simulink model "PrkgClimaPreProcMgr"  (R2022b) (continued)
== system PrkgClimaPreProcMgr == (continued)
  ClngRqrdFromTelmConnGen2_ClngRqrdFromTelmConnGen2/1 -> PrkgClimaPreProcMgr_Runnable_sys/17(ClngRqrdFromTelmConnGen2_ClngRqrdFromTelmConnGen2_read)
  CmptmtObsvrSts_CmptmtObsvrSts/1 -> PrkgClimaPreProcMgr_Runnable_sys/18(CmptmtObsvrSts_CmptmtObsvrSts_read)
  CmptmtSunMtrlTEstimd_CmptmtSunMtrlTEstimd/1 -> PrkgClimaPreProcMgr_Runnable_sys/19(CmptmtSunMtrlTEstimd_CmptmtSunMtrlTEstimd_read)
  DCChrgnHndlSts_DCChrgnHndlSts/1 -> PrkgClimaPreProcMgr_Runnable_sys/20(DCChrgnHndlSts_DCChrgnHndlSts_read)
  DshbTForClima_DshbTForClima/1 -> PrkgClimaPreProcMgr_Runnable_sys/21(DshbTForClima_DshbTForClima_read)
  DshbTForClimaQf_DshbTForClimaQf/1 -> PrkgClimaPreProcMgr_Runnable_sys/22(DshbTForClimaQf_DshbTForClimaQf_read)
  HvacAirTForRowFirstAtVentLeRaw_HvacAirTForRowFirstAtVentLeRaw/1 -> PrkgClimaPreProcMgr_Runnable_sys/23(HvacAirTForRowFirstAtVentLeRaw_HvacAirTForRowFirstAtVentLeRaw_read)
  HvacAirTForRowFirstAtVentRiRaw_HvacAirTForRowFirstAtVentRiRaw/1 -> PrkgClimaPreProcMgr_Runnable_sys/24(HvacAirTForRowFirstAtVentRiRaw_HvacAirTForRowFirstAtVentRiRaw_read)
  MemReadForPrkgClimaPreProcMgr_MemReadForPrkgClimaPreProcMgr/1 -> PrkgClimaPreProcMgr_Runnable_sys/25(MemReadForPrkgClimaPreProcMgr_MemReadForPrkgClimaPreProcMgr_read)
  MemReqStsForPrkgClima_MemReqStsForPrkgClima/1 -> PrkgClimaPreProcMgr_Runnable_sys/26(MemReqStsForPrkgClima_MemReqStsForPrkgClima_read)
  NetReqFromPrkgClimaEveMgr_NetReqFromPrkgClimaEveMgr/1 -> PrkgClimaPreProcMgr_Runnable_sys/27(NetReqFromPrkgClimaEveMgr_NetReqFromPrkgClimaEveMgr_read)
  OnBdChrgrHndlSts_OnBdChrgrHndlSts/1 -> PrkgClimaPreProcMgr_Runnable_sys/28(OnBdChrgrHndlSts_OnBdChrgrHndlSts_read)
  ReqForPrkgClima_ReqForPrkgClima/1 -> PrkgClimaPreProcMgr_Runnable_sys/29(ReqForPrkgClima_ReqForPrkgClima_read)
  ReqForSwtHeatrTypFromActvMgr_ReqForSwtHeatrTypFromActvMgr/1 -> PrkgClimaPreProcMgr_Runnable_sys/30(ReqForSwtHeatrTypFromActvMgr_ReqForSwtHeatrTypFromActvMgr_read)
  ReqForSwtHeatrTypFromEveMgr_ReqForSwtHeatrTypFromEveMgr/1 -> PrkgClimaPreProcMgr_Runnable_sys/31(ReqForSwtHeatrTypFromEveMgr_ReqForSwtHeatrTypFromEveMgr_read)
  RtMaxForPrePrkgClima_RtMaxForPrePrkgClima/1 -> PrkgClimaPreProcMgr_Runnable_sys/32(RtMaxForPrePrkgClima_RtMaxForPrePrkgClima_read)
  SeatHeatDurgClimaEnadConnGen2_SeatHeatDurgClimaEnadConnGen2/1 -> PrkgClimaPreProcMgr_Runnable_sys/33(SeatHeatDurgClimaEnadConnGen2_SeatHeatDurgClimaEnadConnGen2_read)
  SteerWhlHeatgDurgClimEandFromTelmConnGen2_SteerWhlHeatgDurgClimEandFromTelmConnGen2/1 -> PrkgClimaPreProcMgr_Runnable_sys/34(SteerWhlHeatgDurgClimEandFromTelmConnGen2_SteerWhlHeatgDurgClimEandFromTelmConnGen2_read)
  VehModMngtGlbSafe1_Ngcc_VehModMngtGlbSafe1/1 -> PrkgClimaPreProcMgr_Runnable_sys/35(VehModMngtGlbSafe1_Ngcc_VehModMngtGlbSafe1_read)
  WarnForEgyAllwdLimd_WarnForEgyAllwdLimd/1 -> PrkgClimaPreProcMgr_Runnable_sys/36(WarnForEgyAllwdLimd_WarnForEgyAllwdLimd_read)
  WarnForHybWoUForTmr_WarnForHybWoUForTmr/1 -> PrkgClimaPreProcMgr_Runnable_sys/37(WarnForHybWoUForTmr_WarnForHybWoUForTmr_read)
  WarnForHybWoUFromActvMgr_WarnForHybWoUFromActvMgr/1 -> PrkgClimaPreProcMgr_Runnable_sys/38(WarnForHybWoUFromActvMgr_WarnForHybWoUFromActvMgr_read)
  WarnForTmrPreCdngFctNotAvl_WarnForTmrPreCdngFctNotAvl/1 -> PrkgClimaPreProcMgr_Runnable_sys/39(WarnForTmrPreCdngFctNotAvl_WarnForTmrPreCdngFctNotAvl_read)
  BookTrvlClimaActvReq_Ngcc_BookTrvlClimaActvReq/1 -> PrkgClimaPreProcMgr_Runnable_sys/40(BookTrvlClimaActvReq_Ngcc_BookTrvlClimaActvReq_read)
  BookTrvlClimaActvReq_Ngcc_BookTrvlClimaActvReq_IsUpdated/1 -> PrkgClimaPreProcMgr_Runnable_sys/41(BookTrvlClimaActvReq_Ngcc_BookTrvlClimaActvReq_IsUpdated_read)
  CbnOverHeatProtnEna_CbnOverHeatProtnEna/1 -> PrkgClimaPreProcMgr_Runnable_sys/42(CbnOverHeatProtnEna_CbnOverHeatProtnEna_read)
  CbnOverHeatProtnEna_CbnOverHeatProtnEna_IsUpdated/1 -> PrkgClimaPreProcMgr_Runnable_sys/43(CbnOverHeatProtnEna_CbnOverHeatProtnEna_IsUpdated_read)
  CbnOverHeatProtnEnaFromTelm_CbnOverHeatProtnEnaFromTelm/1 -> PrkgClimaPreProcMgr_Runnable_sys/44(CbnOverHeatProtnEnaFromTelm_CbnOverHeatProtnEnaFromTelm_read)
  CbnOverHeatProtnEnaFromTelm_CbnOverHeatProtnEnaFromTelm_IsUpdated/1 -> PrkgClimaPreProcMgr_Runnable_sys/45(CbnOverHeatProtnEnaFromTelm_CbnOverHeatProtnEnaFromTelm_IsUpdated_read)
  CdsClimaOnReq_CdsClimaOnReq/1 -> PrkgClimaPreProcMgr_Runnable_sys/46(CdsClimaOnReq_CdsClimaOnReq_read)
  ClimaRqrdFromHmi_ClimaRqrdFromHmi/1 -> PrkgClimaPreProcMgr_Runnable_sys/47(ClimaRqrdFromHmi_ClimaRqrdFromHmi_read)
  ClimaRqrdFromHmi_ClimaRqrdFromHmi_IsUpdated/1 -> PrkgClimaPreProcMgr_Runnable_sys/48(ClimaRqrdFromHmi_ClimaRqrdFromHmi_IsUpdated_read)
  ClimaTmr_ClimaTmr/1 -> PrkgClimaPreProcMgr_Runnable_sys/49(ClimaTmr_ClimaTmr_read)
  ClimaTmr_ClimaTmr_IsUpdated/1 -> PrkgClimaPreProcMgr_Runnable_sys/50(ClimaTmr_ClimaTmr_IsUpdated_read)
  ClimaTmrStsRqrd_ClimaTmrStsRqrd/1 -> PrkgClimaPreProcMgr_Runnable_sys/51(ClimaTmrStsRqrd_ClimaTmrStsRqrd_read)
  ClimaTmrStsRqrd_ClimaTmrStsRqrd_IsUpdated/1 -> PrkgClimaPreProcMgr_Runnable_sys/52(ClimaTmrStsRqrd_ClimaTmrStsRqrd_IsUpdated_read)
  ClngRqrdFromHmi_ClngRqrdFromHmi/1 -> PrkgClimaPreProcMgr_Runnable_sys/53(ClngRqrdFromHmi_ClngRqrdFromHmi_read)
  ClngRqrdFromHmi_ClngRqrdFromHmi_IsUpdated/1 -> PrkgClimaPreProcMgr_Runnable_sys/54(ClngRqrdFromHmi_ClngRqrdFromHmi_IsUpdated_read)
  ClngRqrdFromTelm_ClngRqrdFromTelm/1 -> PrkgClimaPreProcMgr_Runnable_sys/55(ClngRqrdFromTelm_ClngRqrdFromTelm_read)
  ClngRqrdFromTelm_ClngRqrdFromTelm_IsUpdated/1 -> PrkgClimaPreProcMgr_Runnable_sys/56(ClngRqrdFromTelm_ClngRqrdFromTelm_IsUpdated_read)
  ErsCmdByKey_ErsCmdByKey/1 -> PrkgClimaPreProcMgr_Runnable_sys/57(ErsCmdByKey_ErsCmdByKey_read)
  ErsCmdByKey_ErsCmdByKey_IsUpdated/1 -> PrkgClimaPreProcMgr_Runnable_sys/58(ErsCmdByKey_ErsCmdByKey_IsUpdated_read)
  HeatrPreCdngTyp_HeatrPreCdngTyp/1 -> PrkgClimaPreProcMgr_Runnable_sys/59(HeatrPreCdngTyp_HeatrPreCdngTyp_read)
  HeatrPreCdngTyp_HeatrPreCdngTyp_IsUpdated/1 -> PrkgClimaPreProcMgr_Runnable_sys/60(HeatrPreCdngTyp_HeatrPreCdngTyp_IsUpdated_read)
  IncomingCO2CtrlFromHmi_IncomingCO2CtrlFromHmi/1 -> PrkgClimaPreProcMgr_Runnable_sys/61(IncomingCO2CtrlFromHmi_IncomingCO2CtrlFromHmi_read)
  IncomingCO2CtrlFromHmi_IncomingCO2CtrlFromHmi_IsUpdated/1 -> PrkgClimaPreProcMgr_Runnable_sys/62(IncomingCO2CtrlFromHmi_IncomingCO2CtrlFromHmi_IsUpdated_read)
  RemStrtHvCtrlReq_Ngcc_RemStrtHvCtrlReq/1 -> PrkgClimaPreProcMgr_Runnable_sys/63(RemStrtHvCtrlReq_Ngcc_RemStrtHvCtrlReq_read)
  RemStrtHvCtrlReq_Ngcc_RemStrtHvCtrlReq_IsUpdated/1 -> PrkgClimaPreProcMgr_Runnable_sys/64(RemStrtHvCtrlReq_Ngcc_RemStrtHvCtrlReq_IsUpdated_read)
  RemVentReq_RemVentReq/1 -> PrkgClimaPreProcMgr_Runnable_sys/65(RemVentReq_RemVentReq_read)
  RemVentReq_RemVentReq_IsUpdated/1 -> PrkgClimaPreProcMgr_Runnable_sys/66(RemVentReq_RemVentReq_IsUpdated_read)
  SeatHeatDurgClimaEnad_SeatHeatDurgClimaEnad/1 -> PrkgClimaPreProcMgr_Runnable_sys/67(SeatHeatDurgClimaEnad_SeatHeatDurgClimaEnad_read)
  SeatHeatDurgClimaEnad_SeatHeatDurgClimaEnad_IsUpdated/1 -> PrkgClimaPreProcMgr_Runnable_sys/68(SeatHeatDurgClimaEnad_SeatHeatDurgClimaEnad_IsUpdated_read)
  SeatHeatDurgClimaEnadFromTelm_Ngcc_SeatHeatDurgClimaEnadFromTelm/1 -> PrkgClimaPreProcMgr_Runnable_sys/69(SeatHeatDurgClimaEnadFromTelm_Ngcc_SeatHeatDurgClimaEnadFromTelm_read)
  SeatHeatDurgClimaEnadFromTelm_Ngcc_SeatHeatDurgClimaEnadFromTelm_IsUpdated/1 -> PrkgClimaPreProcMgr_Runnable_sys/70(SeatHeatDurgClimaEnadFromTelm_Ngcc_SeatHeatDurgClimaEnadFromTelm_IsUpdated_read)
  SteerWhlHeatgDurgClimaEnad_SteerWhlHeatgDurgClimaEnad/1 -> PrkgClimaPreProcMgr_Runnable_sys/71(SteerWhlHeatgDurgClimaEnad_SteerWhlHeatgDurgClimaEnad_read)
  SteerWhlHeatgDurgClimaEnad_SteerWhlHeatgDurgClimaEnad_IsUpdated/1 -> PrkgClimaPreProcMgr_Runnable_sys/72(SteerWhlHeatgDurgClimaEnad_SteerWhlHeatgDurgClimaEnad_IsUpdated_read)
  SteerWhlHeatgDurgClimaEnadFromTelm_SteerWhlHeatgDurgClimaEnadFromTelm/1 -> PrkgClimaPreProcMgr_Runnable_sys/73(SteerWhlHeatgDurgClimaEnadFromTelm_SteerWhlHeatgDurgClimaEnadFromTelm_read)
  SteerWhlHeatgDurgClimaEnadFromTelm_SteerWhlHeatgDurgClimaEnadFromTelm_IsUpdated/1 -> PrkgClimaPreProcMgr_Runnable_sys/74(SteerWhlHeatgDurgClimaEnadFromTelm_SteerWhlHeatgDurgClimaEnadFromTelm_IsUpdated_read)
  StrtRemSts1WdStrtRemSts_Ngcc_StrtRemSts1WdStrtRemSts/1 -> PrkgClimaPreProcMgr_Runnable_sys/75(StrtRemSts1WdStrtRemSts_Ngcc_StrtRemSts1WdStrtRemSts_read)
  StrtRemSts1WdStrtRemSts_Ngcc_StrtRemSts1WdStrtRemSts_IsUpdated/1 -> PrkgClimaPreProcMgr_Runnable_sys/76(StrtRemSts1WdStrtRemSts_Ngcc_StrtRemSts1WdStrtRemSts_IsUpdated_read)
  TelmClimaReq_TelmClimaReq/1 -> PrkgClimaPreProcMgr_Runnable_sys/77(TelmClimaReq_TelmClimaReq_read)
  TelmClimaReq_TelmClimaReq_IsUpdated/1 -> PrkgClimaPreProcMgr_Runnable_sys/78(TelmClimaReq_TelmClimaReq_IsUpdated_read)
  TelmClimaTmr_TelmClimaTmr/1 -> PrkgClimaPreProcMgr_Runnable_sys/79(TelmClimaTmr_TelmClimaTmr_read)
  TelmClimaTmr_TelmClimaTmr_IsUpdated/1 -> PrkgClimaPreProcMgr_Runnable_sys/80(TelmClimaTmr_TelmClimaTmr_IsUpdated_read)
  TelmClimaTSet_TelmClimaTSet/1 -> PrkgClimaPreProcMgr_Runnable_sys/81(TelmClimaTSet_TelmClimaTSet_read)
  TelmClimaTSet_TelmClimaTSet_IsUpdated/1 -> PrkgClimaPreProcMgr_Runnable_sys/82(TelmClimaTSet_TelmClimaTSet_IsUpdated_read)
  TelmSeatDrvHeatClimaLvl_TelmSeatDrvHeatClimaLvl/1 -> PrkgClimaPreProcMgr_Runnable_sys/83(TelmSeatDrvHeatClimaLvl_TelmSeatDrvHeatClimaLvl_read)
  TelmSeatDrvHeatClimaLvl_TelmSeatDrvHeatClimaLvl_IsUpdated/1 -> PrkgClimaPreProcMgr_Runnable_sys/84(TelmSeatDrvHeatClimaLvl_TelmSeatDrvHeatClimaLvl_IsUpdated_read)
  TelmSeatDrvVentnClimaLvl_TelmSeatDrvVentnClimaLvl/1 -> PrkgClimaPreProcMgr_Runnable_sys/85(TelmSeatDrvVentnClimaLvl_TelmSeatDrvVentnClimaLvl_read)
  TelmSeatDrvVentnClimaLvl_TelmSeatDrvVentnClimaLvl_IsUpdated/1 -> PrkgClimaPreProcMgr_Runnable_sys/86(TelmSeatDrvVentnClimaLvl_TelmSeatDrvVentnClimaLvl_IsUpdated_read)
  TelmSeatPassHeatClimaLvl_TelmSeatPassHeatClimaLvl/1 -> PrkgClimaPreProcMgr_Runnable_sys/87(TelmSeatPassHeatClimaLvl_TelmSeatPassHeatClimaLvl_read)
  TelmSeatPassHeatClimaLvl_TelmSeatPassHeatClimaLvl_IsUpdated/1 -> PrkgClimaPreProcMgr_Runnable_sys/88(TelmSeatPassHeatClimaLvl_TelmSeatPassHeatClimaLvl_IsUpdated_read)
  TelmSeatPassVentnClimaLvl_TelmSeatPassVentnClimaLvl/1 -> PrkgClimaPreProcMgr_Runnable_sys/89(TelmSeatPassVentnClimaLvl_TelmSeatPassVentnClimaLvl_read)
  TelmSeatPassVentnClimaLvl_TelmSeatPassVentnClimaLvl_IsUpdated/1 -> PrkgClimaPreProcMgr_Runnable_sys/90(TelmSeatPassVentnClimaLvl_TelmSeatPassVentnClimaLvl_IsUpdated_read)
  TelmSeatSecLeHeatClimaLvl_TelmSeatSecLeHeatClimaLvl/1 -> PrkgClimaPreProcMgr_Runnable_sys/91(TelmSeatSecLeHeatClimaLvl_TelmSeatSecLeHeatClimaLvl_read)
  TelmSeatSecLeHeatClimaLvl_TelmSeatSecLeHeatClimaLvl_IsUpdated/1 -> PrkgClimaPreProcMgr_Runnable_sys/92(TelmSeatSecLeHeatClimaLvl_TelmSeatSecLeHeatClimaLvl_IsUpdated_read)
  TelmSeatSecLeVentnClimaLvl_TelmSeatSecLeVentnClimaLvl/1 -> PrkgClimaPreProcMgr_Runnable_sys/93(TelmSeatSecLeVentnClimaLvl_TelmSeatSecLeVentnClimaLvl_read)
  TelmSeatSecLeVentnClimaLvl_TelmSeatSecLeVentnClimaLvl_IsUpdated/1 -> PrkgClimaPreProcMgr_Runnable_sys/94(TelmSeatSecLeVentnClimaLvl_TelmSeatSecLeVentnClimaLvl_IsUpdated_read)
  TelmSeatSecRiHeatClimaLvl_TelmSeatSecRiHeatClimaLvl/1 -> PrkgClimaPreProcMgr_Runnable_sys/95(TelmSeatSecRiHeatClimaLvl_TelmSeatSecRiHeatClimaLvl_read)
  TelmSeatSecRiHeatClimaLvl_TelmSeatSecRiHeatClimaLvl_IsUpdated/1 -> PrkgClimaPreProcMgr_Runnable_sys/96(TelmSeatSecRiHeatClimaLvl_TelmSeatSecRiHeatClimaLvl_IsUpdated_read)
  TelmSeatSecRiVentnClimaLvl_TelmSeatSecRiVentnClimaLvl/1 -> PrkgClimaPreProcMgr_Runnable_sys/97(TelmSeatSecRiVentnClimaLvl_TelmSeatSecRiVentnClimaLvl_read)
  TelmSeatSecRiVentnClimaLvl_TelmSeatSecRiVentnClimaLvl_IsUpdated/1 -> PrkgClimaPreProcMgr_Runnable_sys/98(TelmSeatSecRiVentnClimaLvl_TelmSeatSecRiVentnClimaLvl_IsUpdated_read)
  HMIExtVent_HMIExtVent/1 -> PrkgClimaPreProcMgr_Runnable_sys/99(HMIExtVent_HMIExtVent_read)
  HMIExtVent_HMIExtVent_IsUpdated/1 -> PrkgClimaPreProcMgr_Runnable_sys/100(HMIExtVent_HMIExtVent_IsUpdated_read)
  BookTrvlClimaActvReq2_BookTrvlClimaActvReq2/1 -> PrkgClimaPreProcMgr_Runnable_sys/101(BookTrvlClimaActvReq2_BookTrvlClimaActvReq2_read)
  BookTrvlClimaActvReq2_BookTrvlClimaActvReq2_IsUpdated/1 -> PrkgClimaPreProcMgr_Runnable_sys/102(BookTrvlClimaActvReq2_BookTrvlClimaActvReq2_IsUpdated_read)
  BookTrvlClimaActvReq3_BookTrvlClimaActvReq3/1 -> PrkgClimaPreProcMgr_Runnable_sys/103(BookTrvlClimaActvReq3_BookTrvlClimaActvReq3_read)
  BookTrvlClimaActvReq3_BookTrvlClimaActvReq3_IsUpdated/1 -> PrkgClimaPreProcMgr_Runnable_sys/104(BookTrvlClimaActvReq3_BookTrvlClimaActvReq3_IsUpdated_read)
  BookTrvlClimaActvReq4_BookTrvlClimaActvReq4/1 -> PrkgClimaPreProcMgr_Runnable_sys/105(BookTrvlClimaActvReq4_BookTrvlClimaActvReq4_read)
  BookTrvlClimaActvReq4_BookTrvlClimaActvReq4_IsUpdated/1 -> PrkgClimaPreProcMgr_Runnable_sys/106(BookTrvlClimaActvReq4_BookTrvlClimaActvReq4_IsUpdated_read)
  BookTrvlClimaActvReq5_BookTrvlClimaActvReq5/1 -> PrkgClimaPreProcMgr_Runnable_sys/107(BookTrvlClimaActvReq5_BookTrvlClimaActvReq5_read)
  BookTrvlClimaActvReq5_BookTrvlClimaActvReq5_IsUpdated/1 -> PrkgClimaPreProcMgr_Runnable_sys/108(BookTrvlClimaActvReq5_BookTrvlClimaActvReq5_IsUpdated_read)
  BookTrvlClimaActvReq6_BookTrvlClimaActvReq6/1 -> PrkgClimaPreProcMgr_Runnable_sys/109(BookTrvlClimaActvReq6_BookTrvlClimaActvReq6_read)
  BookTrvlClimaActvReq6_BookTrvlClimaActvReq6_IsUpdated/1 -> PrkgClimaPreProcMgr_Runnable_sys/110(BookTrvlClimaActvReq6_BookTrvlClimaActvReq6_IsUpdated_read)
  BookTrvlClimaActvReq7_BookTrvlClimaActvReq7/1 -> PrkgClimaPreProcMgr_Runnable_sys/111(BookTrvlClimaActvReq7_BookTrvlClimaActvReq7_read)
  BookTrvlClimaActvReq7_BookTrvlClimaActvReq7_IsUpdated/1 -> PrkgClimaPreProcMgr_Runnable_sys/112(BookTrvlClimaActvReq7_BookTrvlClimaActvReq7_IsUpdated_read)
  ClimaWakUpTiDif1_ClimaWakUpTiDif1/1 -> PrkgClimaPreProcMgr_Runnable_sys/113(ClimaWakUpTiDif1_ClimaWakUpTiDif1_read)
  ClimaWakUpTiDif1_ClimaWakUpTiDif1_IsUpdated/1 -> PrkgClimaPreProcMgr_Runnable_sys/114(ClimaWakUpTiDif1_ClimaWakUpTiDif1_IsUpdated_read)
  ClimaWakUpTiDif2_ClimaWakUpTiDif2/1 -> PrkgClimaPreProcMgr_Runnable_sys/115(ClimaWakUpTiDif2_ClimaWakUpTiDif2_read)
  ClimaWakUpTiDif2_ClimaWakUpTiDif2_IsUpdated/1 -> PrkgClimaPreProcMgr_Runnable_sys/116(ClimaWakUpTiDif2_ClimaWakUpTiDif2_IsUpdated_read)
  ClimaWakUpTiDif3_ClimaWakUpTiDif3/1 -> PrkgClimaPreProcMgr_Runnable_sys/117(ClimaWakUpTiDif3_ClimaWakUpTiDif3_read)
  ClimaWakUpTiDif3_ClimaWakUpTiDif3_IsUpdated/1 -> PrkgClimaPreProcMgr_Runnable_sys/118(ClimaWakUpTiDif3_ClimaWakUpTiDif3_IsUpdated_read)
  ClimaWakUpTiDif4_ClimaWakUpTiDif4/1 -> PrkgClimaPreProcMgr_Runnable_sys/119(ClimaWakUpTiDif4_ClimaWakUpTiDif4_read)
  ClimaWakUpTiDif4_ClimaWakUpTiDif4_IsUpdated/1 -> PrkgClimaPreProcMgr_Runnable_sys/120(ClimaWakUpTiDif4_ClimaWakUpTiDif4_IsUpdated_read)
  ClimaWakUpTiDif5_ClimaWakUpTiDif5/1 -> PrkgClimaPreProcMgr_Runnable_sys/121(ClimaWakUpTiDif5_ClimaWakUpTiDif5_read)
  ClimaWakUpTiDif5_ClimaWakUpTiDif5_IsUpdated/1 -> PrkgClimaPreProcMgr_Runnable_sys/122(ClimaWakUpTiDif5_ClimaWakUpTiDif5_IsUpdated_read)
  ClimaWakUpTiDif6_ClimaWakUpTiDif6/1 -> PrkgClimaPreProcMgr_Runnable_sys/123(ClimaWakUpTiDif6_ClimaWakUpTiDif6_read)
  ClimaWakUpTiDif6_ClimaWakUpTiDif6_IsUpdated/1 -> PrkgClimaPreProcMgr_Runnable_sys/124(ClimaWakUpTiDif6_ClimaWakUpTiDif6_IsUpdated_read)
  ClimaWakUpTiDif7_ClimaWakUpTiDif7/1 -> PrkgClimaPreProcMgr_Runnable_sys/125(ClimaWakUpTiDif7_ClimaWakUpTiDif7_read)
  ClimaWakUpTiDif7_ClimaWakUpTiDif7_IsUpdated/1 -> PrkgClimaPreProcMgr_Runnable_sys/126(ClimaWakUpTiDif7_ClimaWakUpTiDif7_IsUpdated_read)
  EngSt1WdSts_Ngcc_EngSt1WdSts/1 -> PrkgClimaPreProcMgr_Runnable_sys/127(EngSt1WdSts_Ngcc_EngSt1WdSts_read)
  HMIExtVentonoffReq_HMIExtVentonoffReq/1 -> PrkgClimaPreProcMgr_Runnable_sys/128(HMIExtVentonoffReq_HMIExtVentonoffReq_read)
  HMIExtVentonoffReq_HMIExtVentonoffReq_IsUpdated/1 -> PrkgClimaPreProcMgr_Runnable_sys/129(HMIExtVentonoffReq_HMIExtVentonoffReq_IsUpdated_read)
  RemStrtExtnTiReq_RemStrtExtnTiReq/1 -> PrkgClimaPreProcMgr_Runnable_sys/130(RemStrtExtnTiReq_RemStrtExtnTiReq_read)
  RemStrtExtnTiReq_RemStrtExtnTiReq_IsUpdated/1 -> PrkgClimaPreProcMgr_Runnable_sys/131(RemStrtExtnTiReq_RemStrtExtnTiReq_IsUpdated_read)
  TelmBookClimaReq_TelmBookClimaReq/1 -> PrkgClimaPreProcMgr_Runnable_sys/132(TelmBookClimaReq_TelmBookClimaReq_read)
  TelmBookClimaReq_TelmBookClimaReq_IsUpdated/1 -> PrkgClimaPreProcMgr_Runnable_sys/133(TelmBookClimaReq_TelmBookClimaReq_IsUpdated_read)
  TelmBookClimaTmr_TelmBookClimaTmr/1 -> PrkgClimaPreProcMgr_Runnable_sys/134(TelmBookClimaTmr_TelmBookClimaTmr_read)
  TelmBookClimaTmr_TelmBookClimaTmr_IsUpdated/1 -> PrkgClimaPreProcMgr_Runnable_sys/135(TelmBookClimaTmr_TelmBookClimaTmr_IsUpdated_read)
  TelmBookSeatHeatClimalReq_TelmBookSeatHeatClimalReq/1 -> PrkgClimaPreProcMgr_Runnable_sys/136(TelmBookSeatHeatClimalReq_TelmBookSeatHeatClimalReq_read)
  TelmBookSeatHeatClimalReq_TelmBookSeatHeatClimalReq_IsUpdated/1 -> PrkgClimaPreProcMgr_Runnable_sys/137(TelmBookSeatHeatClimalReq_TelmBookSeatHeatClimalReq_IsUpdated_read)
  TelmBookSeatVentnClimalReq_TelmBookSeatVentnClimalReq/1 -> PrkgClimaPreProcMgr_Runnable_sys/138(TelmBookSeatVentnClimalReq_TelmBookSeatVentnClimalReq_read)
  TelmBookSeatVentnClimalReq_TelmBookSeatVentnClimalReq_IsUpdated/1 -> PrkgClimaPreProcMgr_Runnable_sys/139(TelmBookSeatVentnClimalReq_TelmBookSeatVentnClimalReq_IsUpdated_read)
  TelmBookSteerWhlHeatClimalReq_TelmBookSteerWhlHeatClimalReq/1 -> PrkgClimaPreProcMgr_Runnable_sys/140(TelmBookSteerWhlHeatClimalReq_TelmBookSteerWhlHeatClimalReq_read)
  TelmBookSteerWhlHeatClimalReq_TelmBookSteerWhlHeatClimalReq_IsUpdated/1 -> PrkgClimaPreProcMgr_Runnable_sys/141(TelmBookSteerWhlHeatClimalReq_TelmBookSteerWhlHeatClimalReq_IsUpdated_read)
  TelmClimaExtVentnLvl_TelmClimaExtVentnLvl/1 -> PrkgClimaPreProcMgr_Runnable_sys/142(TelmClimaExtVentnLvl_TelmClimaExtVentnLvl_read)
  TelmClimaExtVentnLvl_TelmClimaExtVentnLvl_IsUpdated/1 -> PrkgClimaPreProcMgr_Runnable_sys/143(TelmClimaExtVentnLvl_TelmClimaExtVentnLvl_IsUpdated_read)
  TelmClimaExtVentnReq_TelmClimaExtVentnReq/1 -> PrkgClimaPreProcMgr_Runnable_sys/144(TelmClimaExtVentnReq_TelmClimaExtVentnReq_read)
  TelmClimaExtVentnReq_TelmClimaExtVentnReq_IsUpdated/1 -> PrkgClimaPreProcMgr_Runnable_sys/145(TelmClimaExtVentnReq_TelmClimaExtVentnReq_IsUpdated_read)
  TelmClimaExtVentnTempSet_TelmClimaExtVentnTempSet/1 -> PrkgClimaPreProcMgr_Runnable_sys/146(TelmClimaExtVentnTempSet_TelmClimaExtVentnTempSet_read)
  TelmClimaExtVentnTempSet_TelmClimaExtVentnTempSet_IsUpdated/1 -> PrkgClimaPreProcMgr_Runnable_sys/147(TelmClimaExtVentnTempSet_TelmClimaExtVentnTempSet_IsUpdated_read)
  TelmDefrostReq_TelmDefrostReq/1 -> PrkgClimaPreProcMgr_Runnable_sys/148(TelmDefrostReq_TelmDefrostReq_read)
  TelmDefrostReq_TelmDefrostReq_IsUpdated/1 -> PrkgClimaPreProcMgr_Runnable_sys/149(TelmDefrostReq_TelmDefrostReq_IsUpdated_read)
  TelmOxygenReq_TelmOxygenReq/1 -> PrkgClimaPreProcMgr_Runnable_sys/150(TelmOxygenReq_TelmOxygenReq_read)
  TelmOxygenReq_TelmOxygenReq_IsUpdated/1 -> PrkgClimaPreProcMgr_Runnable_sys/151(TelmOxygenReq_TelmOxygenReq_IsUpdated_read)
  TelmSeatThrdLeHeatClimaLvl_TelmSeatThrdLeHeatClimaLvl/1 -> PrkgClimaPreProcMgr_Runnable_sys/152(TelmSeatThrdLeHeatClimaLvl_TelmSeatThrdLeHeatClimaLvl_read)
  TelmSeatThrdLeHeatClimaLvl_TelmSeatThrdLeHeatClimaLvl_IsUpdated/1 -> PrkgClimaPreProcMgr_Runnable_sys/153(TelmSeatThrdLeHeatClimaLvl_TelmSeatThrdLeHeatClimaLvl_IsUpdated_read)
  TelmSeatThrdLeVentnClimaLvl_TelmSeatThrdLeVentnClimaLvl/1 -> PrkgClimaPreProcMgr_Runnable_sys/154(TelmSeatThrdLeVentnClimaLvl_TelmSeatThrdLeVentnClimaLvl_read)
  TelmSeatThrdLeVentnClimaLvl_TelmSeatThrdLeVentnClimaLvl_IsUpdated/1 -> PrkgClimaPreProcMgr_Runnable_sys/155(TelmSeatThrdLeVentnClimaLvl_TelmSeatThrdLeVentnClimaLvl_IsUpdated_read)
  TelmSeatThrdRiHeatClimaLvl_TelmSeatThrdRiHeatClimaLvl/1 -> PrkgClimaPreProcMgr_Runnable_sys/156(TelmSeatThrdRiHeatClimaLvl_TelmSeatThrdRiHeatClimaLvl_read)
  TelmSeatThrdRiHeatClimaLvl_TelmSeatThrdRiHeatClimaLvl_IsUpdated/1 -> PrkgClimaPreProcMgr_Runnable_sys/157(TelmSeatThrdRiHeatClimaLvl_TelmSeatThrdRiHeatClimaLvl_IsUpdated_read)
  TelmSeatThrdRiVentnClimaLvl_TelmSeatThrdRiVentnClimaLvl/1 -> PrkgClimaPreProcMgr_Runnable_sys/158(TelmSeatThrdRiVentnClimaLvl_TelmSeatThrdRiVentnClimaLvl_read)
  TelmSeatThrdRiVentnClimaLvl_TelmSeatThrdRiVentnClimaLvl_IsUpdated/1 -> PrkgClimaPreProcMgr_Runnable_sys/159(TelmSeatThrdRiVentnClimaLvl_TelmSeatThrdRiVentnClimaLvl_IsUpdated_read)
  PrkgClimaPreProcMgr_Runnable_sys/1(BookTrvlClimaActvReq2FromExSrc_BookTrvlClimaActvReq2FromExSrc_write) -> BookTrvlClimaActvReq2FromExSrc_BookTrvlClimaActvReq2FromExSrc/1
  PrkgClimaPreProcMgr_Runnable_sys/2(BookTrvlClimaActvReq3FromExSrc_BookTrvlClimaActvReq3FromExSrc_write) -> BookTrvlClimaActvReq3FromExSrc_BookTrvlClimaActvReq3FromExSrc/1
  PrkgClimaPreProcMgr_Runnable_sys/3(BookTrvlClimaActvReq4FromExSrc_BookTrvlClimaActvReq4FromExSrc_write) -> BookTrvlClimaActvReq4FromExSrc_BookTrvlClimaActvReq4FromExSrc/1
  PrkgClimaPreProcMgr_Runnable_sys/4(BookTrvlClimaActvReq5FromExSrc_BookTrvlClimaActvReq5FromExSrc_write) -> BookTrvlClimaActvReq5FromExSrc_BookTrvlClimaActvReq5FromExSrc/1
  PrkgClimaPreProcMgr_Runnable_sys/5(BookTrvlClimaActvReq6FromExSrc_BookTrvlClimaActvReq6FromExSrc_write) -> BookTrvlClimaActvReq6FromExSrc_BookTrvlClimaActvReq6FromExSrc/1
  PrkgClimaPreProcMgr_Runnable_sys/6(BookTrvlClimaActvReq7FromExSrc_BookTrvlClimaActvReq7FromExSrc_write) -> BookTrvlClimaActvReq7FromExSrc_BookTrvlClimaActvReq7FromExSrc/1
  PrkgClimaPreProcMgr_Runnable_sys/7(ClimaWakUpTiDif1FromExSrc_ClimaWakUpTiDif1FromExSrc_write) -> ClimaWakUpTiDif1FromExSrc_ClimaWakUpTiDif1FromExSrc/1
  PrkgClimaPreProcMgr_Runnable_sys/8(ClimaWakUpTiDif2FromExSrc_ClimaWakUpTiDif2FromExSrc_write) -> ClimaWakUpTiDif2FromExSrc_ClimaWakUpTiDif2FromExSrc/1
  PrkgClimaPreProcMgr_Runnable_sys/9(ClimaWakUpTiDif3FromExSrc_ClimaWakUpTiDif3FromExSrc_write) -> ClimaWakUpTiDif3FromExSrc_ClimaWakUpTiDif3FromExSrc/1
  PrkgClimaPreProcMgr_Runnable_sys/10(ClimaWakUpTiDif4FromExSrc_ClimaWakUpTiDif4FromExSrc_write) -> ClimaWakUpTiDif4FromExSrc_ClimaWakUpTiDif4FromExSrc/1
  PrkgClimaPreProcMgr_Runnable_sys/11(ClimaWakUpTiDif5FromExSrc_ClimaWakUpTiDif5FromExSrc_write) -> ClimaWakUpTiDif5FromExSrc_ClimaWakUpTiDif5FromExSrc/1
  PrkgClimaPreProcMgr_Runnable_sys/12(ClimaWakUpTiDif6FromExSrc_ClimaWakUpTiDif6FromExSrc_write) -> ClimaWakUpTiDif6FromExSrc_ClimaWakUpTiDif6FromExSrc/1
  PrkgClimaPreProcMgr_Runnable_sys/13(ClimaWakUpTiDif7FromExSrc_ClimaWakUpTiDif7FromExSrc_write) -> ClimaWakUpTiDif7FromExSrc_ClimaWakUpTiDif7FromExSrc/1
  PrkgClimaPreProcMgr_Runnable_sys/14(EngSt1WdStsFromExsrc_EngSt1WdStsFromExsrc_write) -> EngSt1WdStsFromExsrc_EngSt1WdStsFromExsrc/1
  PrkgClimaPreProcMgr_Runnable_sys/15(RemClimaHvRspn_RemClimaHvRspn_write) -> RemClimaHvRspn_RemClimaHvRspn/1
  PrkgClimaPreProcMgr_Runnable_sys/16(TelmOxygenReqFromExSrc_TelmOxygenReqFromExSrc_write) -> TelmOxygenReqFromExSrc_TelmOxygenReqFromExSrc/1
  PrkgClimaPreProcMgr_Runnable_sys/17(TelmSeatThrdLeHeatReqFromExsrc_TelmSeatThrdLeHeatReqFromExsrc_write) -> TelmSeatThrdLeHeatReqFromExsrc_TelmSeatThrdLeHeatReqFromExsrc/1
  PrkgClimaPreProcMgr_Runnable_sys/18(TelmSeatThrdLeVentnReqFromExsrc_TelmSeatThrdLeVentnReqFromExsrc_write) -> TelmSeatThrdLeVentnReqFromExsrc_TelmSeatThrdLeVentnReqFromExsrc/1
  PrkgClimaPreProcMgr_Runnable_sys/19(TelmSeatThrdRiHeatReqFromExsrc_TelmSeatThrdRiHeatReqFromExsrc_write) -> TelmSeatThrdRiHeatReqFromExsrc_TelmSeatThrdRiHeatReqFromExsrc/1
  PrkgClimaPreProcMgr_Runnable_sys/20(TelmSeatThrdRiVentnReqFromExsrc_TelmSeatThrdRiVentnReqFromExsrc_write) -> TelmSeatThrdRiVentnReqFromExsrc_TelmSeatThrdRiVentnReqFromExsrc/1
  PrkgClimaPreProcMgr_Runnable_sys/21(BookTrvlClimaActvReqfromExsrc_BookTrvlClimaActvReqfromExsrc_write) -> BookTrvlClimaActvReqfromExsrc_BookTrvlClimaActvReqfromExsrc/1
  PrkgClimaPreProcMgr_Runnable_sys/22(CdsClimaOnReqfromExsrc_CdsClimaOnReqfromExsrc_write) -> CdsClimaOnReqfromExsrc_CdsClimaOnReqfromExsrc/1
  PrkgClimaPreProcMgr_Runnable_sys/23(ClimaOvrHeatProRspn_ClimaOvrHeatProRspn_write) -> ClimaOvrHeatProRspn_ClimaOvrHeatProRspn/1
  PrkgClimaPreProcMgr_Runnable_sys/24(ClimaOvrHeatReqFromExrsc_ClimaOvrHeatReqFromExrsc_write) -> ClimaOvrHeatReqFromExrsc_ClimaOvrHeatReqFromExrsc/1
  PrkgClimaPreProcMgr_Runnable_sys/25(ClimaStrtTiDly_ClimaStrtTiDly_write) -> ClimaStrtTiDly_ClimaStrtTiDly/1
  PrkgClimaPreProcMgr_Runnable_sys/26(ClimaTmrSts_ClimaTmrSts_write) -> ClimaTmrSts_ClimaTmrSts/1
  PrkgClimaPreProcMgr_Runnable_sys/27(DshbTForPrkgClima_DshbTForPrkgClima_write) -> DshbTForPrkgClima_DshbTForPrkgClima/1
  PrkgClimaPreProcMgr_Runnable_sys/28(ErsCmdByKeyFromExsrc_ErsCmdByKeyFromExsrc_write) -> ErsCmdByKeyFromExsrc_ErsCmdByKeyFromExsrc/1
  PrkgClimaPreProcMgr_Runnable_sys/29(ErsRunTimeFromExtSrc_ErsRunTimeFromExtSrc_write) -> ErsRunTimeFromExtSrc_ErsRunTimeFromExtSrc/1
  PrkgClimaPreProcMgr_Runnable_sys/30(HeatrElecNotAvlForTmr_HeatrElecNotAvlForTmr_write) -> HeatrElecNotAvlForTmr_HeatrElecNotAvlForTmr/1
  PrkgClimaPreProcMgr_Runnable_sys/31(HeatrTypSetgForPrkgClima_HeatrTypSetgForPrkgClima_write) -> HeatrTypSetgForPrkgClima_HeatrTypSetgForPrkgClima/1
  PrkgClimaPreProcMgr_Runnable_sys/32(HeatUpRqrdForPrkgClima_HeatUpRqrdForPrkgClima_write) -> HeatUpRqrdForPrkgClima_HeatUpRqrdForPrkgClima/1
  PrkgClimaPreProcMgr_Runnable_sys/33(HMIExtVentFanVolReqFromExtSrc_HMIExtVentFanVolReqFromExtSrc_write) -> HMIExtVentFanVolReqFromExtSrc_HMIExtVentFanVolReqFromExtSrc/1
  PrkgClimaPreProcMgr_Runnable_sys/34(HMIExtVentonoffReqFromExtSrc_HMIExtVentonoffReqFromExtSrc_write) -> HMIExtVentonoffReqFromExtSrc_HMIExtVentonoffReqFromExtSrc/1
  PrkgClimaPreProcMgr_Runnable_sys/35(HMIExtVentTSpReqFromExtSrc_HMIExtVentTSpReqFromExtSrc_write) -> HMIExtVentTSpReqFromExtSrc_HMIExtVentTSpReqFromExtSrc/1
  PrkgClimaPreProcMgr_Runnable_sys/36(HMIExtVentTStsReqFromExtSrc_HMIExtVentTStsReqFromExtSrc_write) -> HMIExtVentTStsReqFromExtSrc_HMIExtVentTStsReqFromExtSrc/1
  PrkgClimaPreProcMgr_Runnable_sys/37(HvSysCnctWoU_HvSysCnctWoU_write) -> HvSysCnctWoU_HvSysCnctWoU/1
  PrkgClimaPreProcMgr_Runnable_sys/38(HvSysWoU_HvSysWoU_write) -> HvSysWoU_HvSysWoU/1
  PrkgClimaPreProcMgr_Runnable_sys/39(IntCo2fromExsrc_IntCo2fromExsrc_write) -> IntCo2fromExsrc_IntCo2fromExsrc/1
  PrkgClimaPreProcMgr_Runnable_sys/40(MemSaveFlgForPrkgClimaPreProcMgr_MemSaveFlgForPrkgClimaPreProcMgr_write) -> MemSaveFlgForPrkgClimaPreProcMgr_MemSaveFlgForPrkgClimaPreProcMgr/1
  MemWrForPrkgClimaPreProcMgr_MemWrForPrkgClimaPreProcMgr_merge/1 -> MemWrForPrkgClimaPreProcMgr_MemWrForPrkgClimaPreProcMgr/1
  PrkgClimaPreProcMgr_Runnable_sys/42(PreClngRqrdFromExtSrc_PreClngRqrdFromExtSrc_write) -> PreClngRqrdFromExtSrc_PreClngRqrdFromExtSrc/1
  PrkgClimaPreProcMgr_Runnable_sys/43(PrkgClimaRqrdImdtFromExtSrc_PrkgClimaRqrdImdtFromExtSrc_write) -> PrkgClimaRqrdImdtFromExtSrc_PrkgClimaRqrdImdtFromExtSrc/1
  PrkgClimaPreProcMgr_Runnable_sys/44(RemClimaReqFromExtSrc_RemClimaReqFromExtSrc_write) -> RemClimaReqFromExtSrc_RemClimaReqFromExtSrc/1
  PrkgClimaPreProcMgr_Runnable_sys/45(RemStrtExtnTiReqFromExtSrc_RemStrtExtnTiReqFromExtSrc_write) -> RemStrtExtnTiReqFromExtSrc_RemStrtExtnTiReqFromExtSrc/1
  PrkgClimaPreProcMgr_Runnable_sys/46(RemStrtHvCtrlReqFromExtSrc_RemStrtHvCtrlReqFromExtSrc_write) -> RemStrtHvCtrlReqFromExtSrc_RemStrtHvCtrlReqFromExtSrc/1
  PrkgClimaPreProcMgr_Runnable_sys/47(RemVentReqfromExsrc_RemVentReqfromExsrc_write) -> RemVentReqfromExsrc_RemVentReqfromExsrc/1
  PrkgClimaPreProcMgr_Runnable_sys/48(SeatHeatgEnadFromHmi_SeatHeatgEnadFromHmi_write) -> SeatHeatgEnadFromHmi_SeatHeatgEnadFromHmi/1
  PrkgClimaPreProcMgr_Runnable_sys/49(SeatHeatgEnadFromTelm_SeatHeatgEnadFromTelm_write) -> SeatHeatgEnadFromTelm_SeatHeatgEnadFromTelm/1
  PrkgClimaPreProcMgr_Runnable_sys/50(SnsrQfOkForPrkgClima_SnsrQfOkForPrkgClima_write) -> SnsrQfOkForPrkgClima_SnsrQfOkForPrkgClima/1
  PrkgClimaPreProcMgr_Runnable_sys/51(SteerWhlHeatgDurgClimaEnadFromExsrc_SteerWhlHeatgDurgClimaEnadFromExsrc_write) -> SteerWhlHeatgDurgClimaEnadFromExsrc_SteerWhlHeatgDurgClimaEnadFromExsrc/1
  PrkgClimaPreProcMgr_Runnable_sys/52(SteerWhlHeatgEnadFromHmi_SteerWhlHeatgEnadFromHmi_write) -> SteerWhlHeatgEnadFromHmi_SteerWhlHeatgEnadFromHmi/1
  PrkgClimaPreProcMgr_Runnable_sys/53(SteerWhlHeatgEnadFromTelm_SteerWhlHeatgEnadFromTelm_write) -> SteerWhlHeatgEnadFromTelm_SteerWhlHeatgEnadFromTelm/1
  PrkgClimaPreProcMgr_Runnable_sys/54(StrtRemStsFromExsrc_StrtRemStsFromExsrc_write) -> StrtRemStsFromExsrc_StrtRemStsFromExsrc/1
  PrkgClimaPreProcMgr_Runnable_sys/55(TelmBookClimaReqHmiCmptmtTSpSpclFromExtSrc_TelmBookClimaReqHmiCmptmtTSpSpclFromExtSrc_write) -> TelmBookClimaReqHmiCmptmtTSpSpclFromExtSrc_TelmBookClimaReqHmiCmptmtTSpSpclFromExtSrc/1
  PrkgClimaPreProcMgr_Runnable_sys/56(TelmBookClimaReqOnOffNoReqFromExtSrc_TelmBookClimaReqOnOffNoReqFromExtSrc_write) -> TelmBookClimaReqOnOffNoReqFromExtSrc_TelmBookClimaReqOnOffNoReqFromExtSrc/1
  PrkgClimaPreProcMgr_Runnable_sys/57(TelmBookClimaReqTempRangeFromExtSrc_TelmBookClimaReqTempRangeFromExtSrc_write) -> TelmBookClimaReqTempRangeFromExtSrc_TelmBookClimaReqTempRangeFromExtSrc/1
  PrkgClimaPreProcMgr_Runnable_sys/58(TelmBookClimaTmrFromExtSrc_TelmBookClimaTmrFromExtSrc_write) -> TelmBookClimaTmrFromExtSrc_TelmBookClimaTmrFromExtSrc/1
  PrkgClimaPreProcMgr_Runnable_sys/59(TelmBookSteerWhlHeatClimalReqFromExtSrc_TelmBookSteerWhlHeatClimalReqFromExtSrc_write) -> TelmBookSteerWhlHeatClimalReqFromExtSrc_TelmBookSteerWhlHeatClimalReqFromExtSrc/1
  PrkgClimaPreProcMgr_Runnable_sys/60(TelmClimaExtVentnLvlFromExtSrc_TelmClimaExtVentnLvlFromExtSrc_write) -> TelmClimaExtVentnLvlFromExtSrc_TelmClimaExtVentnLvlFromExtSrc/1
  PrkgClimaPreProcMgr_Runnable_sys/61(TelmClimaExtVentnReqFromExtSrc_TelmClimaExtVentnReqFromExtSrc_write) -> TelmClimaExtVentnReqFromExtSrc_TelmClimaExtVentnReqFromExtSrc/1
  PrkgClimaPreProcMgr_Runnable_sys/62(TelmClimaExtVentnTempRangeFromExtSrc_TelmClimaExtVentnTempRangeFromExtSrc_write) -> TelmClimaExtVentnTempRangeFromExtSrc_TelmClimaExtVentnTempRangeFromExtSrc/1
  PrkgClimaPreProcMgr_Runnable_sys/63(TelmClimaExtVentnTSpSpclFromExtSrc_TelmClimaExtVentnTSpSpclFromExtSrc_write) -> TelmClimaExtVentnTSpSpclFromExtSrc_TelmClimaExtVentnTSpSpclFromExtSrc/1
  PrkgClimaPreProcMgr_Runnable_sys/64(TelmClimaTmrFromExtSrc_TelmClimaTmrFromExtSrc_write) -> TelmClimaTmrFromExtSrc_TelmClimaTmrFromExtSrc/1
  PrkgClimaPreProcMgr_Runnable_sys/65(TelmSeatDrvrHeatReqFromExsrc_TelmSeatDrvrHeatReqFromExsrc_write) -> TelmSeatDrvrHeatReqFromExsrc_TelmSeatDrvrHeatReqFromExsrc/1
  PrkgClimaPreProcMgr_Runnable_sys/66(TelmSeatDrvVentnReqFromExsrc_TelmSeatDrvVentnReqFromExsrc_write) -> TelmSeatDrvVentnReqFromExsrc_TelmSeatDrvVentnReqFromExsrc/1
  PrkgClimaPreProcMgr_Runnable_sys/67(TelmSeatPassHeatReqFromExsrc_TelmSeatPassHeatReqFromExsrc_write) -> TelmSeatPassHeatReqFromExsrc_TelmSeatPassHeatReqFromExsrc/1
  PrkgClimaPreProcMgr_Runnable_sys/68(TelmSeatPassVentnReqFromExsrc_TelmSeatPassVentnReqFromExsrc_write) -> TelmSeatPassVentnReqFromExsrc_TelmSeatPassVentnReqFromExsrc/1
  PrkgClimaPreProcMgr_Runnable_sys/69(TelmSeatSecLeHeatReqFromExsrc_TelmSeatSecLeHeatReqFromExsrc_write) -> TelmSeatSecLeHeatReqFromExsrc_TelmSeatSecLeHeatReqFromExsrc/1
  PrkgClimaPreProcMgr_Runnable_sys/70(TelmSeatSecLeVentnReqFromExsrc_TelmSeatSecLeVentnReqFromExsrc_write) -> TelmSeatSecLeVentnReqFromExsrc_TelmSeatSecLeVentnReqFromExsrc/1
  PrkgClimaPreProcMgr_Runnable_sys/71(TelmSeatSecRiHeatReqFromExsrc_TelmSeatSecRiHeatReqFromExsrc_write) -> TelmSeatSecRiHeatReqFromExsrc_TelmSeatSecRiHeatReqFromExsrc/1
  PrkgClimaPreProcMgr_Runnable_sys/72(TelmSeatSecRiVentnReqFromExsrc_TelmSeatSecRiVentnReqFromExsrc_write) -> TelmSeatSecRiVentnReqFromExsrc_TelmSeatSecRiVentnReqFromExsrc/1
  PrkgClimaPreProcMgr_Runnable_sys/73(TmrForPrkgClima_TmrForPrkgClima_write) -> TmrForPrkgClima_TmrForPrkgClima/1
  PrkgClimaPreProcMgr_Runnable_sys/74(WarnForEgyAllwdLimdDly_WarnForEgyAllwdLimdDly_write) -> WarnForEgyAllwdLimdDly_WarnForEgyAllwdLimdDly/1
  PrkgClimaPreProcMgr_Runnable_sys/75(TelmBookSeatHeatClimalReqDrvHeatClimaLvlFromExtSrc_TelmBookSeatHeatClimalReqDrvHeatClimaLvlFromExtSrc_write) -> TelmBookSeatHeatClimalReqDrvHeatClimaLvlFromExtSrc_TelmBookSeatHeatClimalReqDrvHeatClimaLvlFromExtSrc/1
  PrkgClimaPreProcMgr_Runnable_sys/76(TelmBookSeatHeatClimalReqPassHeatClimaLvlFromExtSrc_TelmBookSeatHeatClimalReqPassHeatClimaLvlFromExtSrc_write) -> TelmBookSeatHeatClimalReqPassHeatClimaLvlFromExtSrc_TelmBookSeatHeatClimalReqPassHeatClimaLvlFromExtSrc/1
  PrkgClimaPreProcMgr_Runnable_sys/77(TelmBookSeatHeatClimalReqSecLeHeatClimaLvlFromExtSrc_TelmBookSeatHeatClimalReqSecLeHeatClimaLvlFromExtSrc_write) -> TelmBookSeatHeatClimalReqSecLeHeatClimaLvlFromExtSrc_TelmBookSeatHeatClimalReqSecLeHeatClimaLvlFromExtSrc/1
  PrkgClimaPreProcMgr_Runnable_sys/78(TelmBookSeatHeatClimalReqSecRiHeatClimaLvlFromExtSrc_TelmBookSeatHeatClimalReqSecRiHeatClimaLvlFromExtSrc_write) -> TelmBookSeatHeatClimalReqSecRiHeatClimaLvlFromExtSrc_TelmBookSeatHeatClimalReqSecRiHeatClimaLvlFromExtSrc/1
  PrkgClimaPreProcMgr_Runnable_sys/79(TelmBookSeatHeatClimalReqThrdLeHeatClimaLvlFromExtSrc_TelmBookSeatHeatClimalReqThrdLeHeatClimaLvlFromExtSrc_write) -> TelmBookSeatHeatClimalReqThrdLeHeatClimaLvlFromExtSrc_TelmBookSeatHeatClimalReqThrdLeHeatClimaLvlFromExtSrc/1
  PrkgClimaPreProcMgr_Runnable_sys/80(TelmBookSeatHeatClimalReqThrdRiHeatClimaLvlFromExtSrc_TelmBookSeatHeatClimalReqThrdRiHeatClimaLvlFromExtSrc_write) -> TelmBookSeatHeatClimalReqThrdRiHeatClimaLvlFromExtSrc_TelmBookSeatHeatClimalReqThrdRiHeatClimaLvlFromExtSrc/1
  PrkgClimaPreProcMgr_Runnable_sys/81(TelmBookSeatVentnClimalReqDrvVentnClimaLvlFromExtSrc_TelmBookSeatVentnClimalReqDrvVentnClimaLvlFromExtSrc_write) -> TelmBookSeatVentnClimalReqDrvVentnClimaLvlFromExtSrc_TelmBookSeatVentnClimalReqDrvVentnClimaLvlFromExtSrc/1
  PrkgClimaPreProcMgr_Runnable_sys/82(TelmBookSeatVentnClimalReqPassVentnClimaLvlFromExtSrc_TelmBookSeatVentnClimalReqPassVentnClimaLvlFromExtSrc_write) -> TelmBookSeatVentnClimalReqPassVentnClimaLvlFromExtSrc_TelmBookSeatVentnClimalReqPassVentnClimaLvlFromExtSrc/1
  PrkgClimaPreProcMgr_Runnable_sys/83(TelmBookSeatVentnClimalReqSecLeVentnClimaLvlFromExtSrc_TelmBookSeatVentnClimalReqSecLeVentnClimaLvlFromExtSrc_write) -> TelmBookSeatVentnClimalReqSecLeVentnClimaLvlFromExtSrc_TelmBookSeatVentnClimalReqSecLeVentnClimaLvlFromExtSrc/1
  PrkgClimaPreProcMgr_Runnable_sys/84(TelmBookSeatVentnClimalReqSecRiVentnClimaLvlFromExtSrc_TelmBookSeatVentnClimalReqSecRiVentnClimaLvlFromExtSrc_write) -> TelmBookSeatVentnClimalReqSecRiVentnClimaLvlFromExtSrc_TelmBookSeatVentnClimalReqSecRiVentnClimaLvlFromExtSrc/1
  PrkgClimaPreProcMgr_Runnable_sys/85(TelmBookSeatVentnClimalReqThrdLeVentnClimaLvlFromExtSrc_TelmBookSeatVentnClimalReqThrdLeVentnClimaLvlFromExtSrc_write) -> TelmBookSeatVentnClimalReqThrdLeVentnClimaLvlFromExtSrc_TelmBookSeatVentnClimalReqThrdLeVentnClimaLvlFromExtSrc/1
  PrkgClimaPreProcMgr_Runnable_sys/86(TelmBookSeatVentnClimalReqThrdRiVentnClimaLvlFromExtSrc_TelmBookSeatVentnClimalReqThrdRiVentnClimaLvlFromExtSrc_write) -> TelmBookSeatVentnClimalReqThrdRiVentnClimaLvlFromExtSrc_TelmBookSeatVentnClimalReqThrdRiVentnClimaLvlFromExtSrc/1
  PrkgClimaPreProcMgr_Runnable_sys/87(TelmDefrostReqFromExsrc_TelmDefrostReqFromExsrc_write) -> TelmDefrostReqFromExsrc_TelmDefrostReqFromExsrc/1
  PrkgClimaPreProcMgr_Runnable_sys/88(TempRangeFromExtSrc_TempRangeFromExtSrc_write) -> TempRangeFromExtSrc_TempRangeFromExtSrc/1
  PrkgClimaPreProcMgr_Runnable_sys/41(MemWrForPrkgClimaPreProcMgr_MemWrForPrkgClimaPreProcMgr_write) -> [MemWrForPrkgClimaPreProcMgr_MemWrForPrkgClimaP_e87563ef2719b2bb]
  [MemWrForPrkgClimaPreProcMgr_MemWrForPrkgClimaP_e87563ef2719b2bb] -> MemWrForPrkgClimaPreProcMgr_MemWrForPrkgClimaPreProcMgr_merge/2
  PrkgClimaPreProcMgr_Init/1(MemWrForPrkgClimaPreProcMgr_MemWrForPrkgClimaPreProcMgr_write) -> [MemWrForPrkgClimaPreProcMgr_MemWrForPrkgClimaP_a490fd5a3a37a0d8]
  [MemWrForPrkgClimaPreProcMgr_MemWrForPrkgClimaP_a490fd5a3a37a0d8] -> MemWrForPrkgClimaPreProcMgr_MemWrForPrkgClimaPreProcMgr_merge/1
  PrkgClimaPreProcMgr_Runnable/1 -> PrkgClimaPreProcMgr_Runnable_sys/Trigger
